simulink model "controller_observer_AllAgents_v5"  (Simulink 8.4) (continued)
== system controller_observer_AllAgents_v5/Controller == (continued)
  [TzCornerClass2Pod1] -> CornerClass2Pod1_Cooling_Mux/1
  [Tst_Cooling] -> CornerClass2Pod1_Cooling_Mux/5
  [Tamb] -> CornerClass2Pod1_Cooling_Mux/2
  CornerClass2Pod1_Cooling/1(Out1) -> [mcCornerClass2Pod1_Cooling]
  [TzNeighborCornerClass2Pod1] -> CornerClass2Pod1_Cooling_Mux/6
  CornerClass2Pod1_Cooling/3(Out3) -> [TstRefCornerClass2Pod1_Cooling]
  [TcCornerClass2Pod1_Cooling] -> CornerClass2Pod1_Cooling_Mux/3
  CornerClass2Pod1_Cooling_Mux/1 -> CornerClass2Pod1_Cooling/1(In1)
  [TsaCornerClass2Pod1] -> CornerClass2Pod1_Cooling_Mux/4
  CornerClass2Pod1_Cooling/2(Out2) -> [Mc_Tst_Tc_CornerClass2Pod1_Cooling]
  [TzCornerClass1Pod2] -> CornerClass1Pod2_Cooling_Mux/1
  [Tst_Cooling] -> CornerClass1Pod2_Cooling_Mux/5
  [Tamb] -> CornerClass1Pod2_Cooling_Mux/2
  CornerClass1Pod2_Cooling/1(Out1) -> [mcCornerClass1Pod2_Cooling]
  [TzNeighborCornerClass1Pod2] -> CornerClass1Pod2_Cooling_Mux/6
  CornerClass1Pod2_Cooling/3(Out3) -> [TstRefCornerClass1Pod2_Cooling]
  [TcCornerClass1Pod2_Cooling] -> CornerClass1Pod2_Cooling_Mux/3
  [TsaCornerClass1Pod2] -> CornerClass1Pod2_Cooling_Mux/4
  CornerClass1Pod2_Cooling/2(Out2) -> [Mc_Tst_Tc_CornerClass1Pod2_Cooling]
  [TzCornerClass2Pod2] -> CornerClass2Pod2_Cooling_Mux/1
  [Tst_Cooling] -> CornerClass2Pod2_Cooling_Mux/5
  [Tamb] -> CornerClass2Pod2_Cooling_Mux/2
  CornerClass2Pod2_Cooling/1(Out1) -> [mcCornerClass2Pod2_Cooling]
  [TzNeighborCornerClass2Pod2] -> CornerClass2Pod2_Cooling_Mux/6
  CornerClass2Pod2_Cooling/3(Out3) -> [TstRefCornerClass2Pod2_Cooling]
  [TcCornerClass2Pod2_Cooling] -> CornerClass2Pod2_Cooling_Mux/3
  CornerClass2Pod2_Cooling_Mux/1 -> CornerClass2Pod2_Cooling/1(In1)
  [TsaCornerClass2Pod2] -> CornerClass2Pod2_Cooling_Mux/4
  CornerClass2Pod2_Cooling/2(Out2) -> [Mc_Tst_Tc_CornerClass2Pod2_Cooling]
  [TzCornerClass1Pod3] -> CornerClass1Pod3_Cooling_Mux/1
  [Tst_Cooling] -> CornerClass1Pod3_Cooling_Mux/5
  [Tamb] -> CornerClass1Pod3_Cooling_Mux/2
  CornerClass1Pod3_Cooling/1(Out1) -> [mcCornerClass1Pod3_Cooling]
  [TzNeighborCornerClass1Pod3] -> CornerClass1Pod3_Cooling_Mux/6
  CornerClass1Pod3_Cooling/3(Out3) -> [TstRefCornerClass1Pod3_Cooling]
  [TcCornerClass1Pod3_Cooling] -> CornerClass1Pod3_Cooling_Mux/3
  CornerClass1Pod3_Cooling_Mux/1 -> CornerClass1Pod3_Cooling/1(In1)
  [TsaCornerClass1Pod3] -> CornerClass1Pod3_Cooling_Mux/4
  CornerClass1Pod3_Cooling/2(Out2) -> [Mc_Tst_Tc_CornerClass1Pod3_Cooling]
  [TzCornerClass2Pod3] -> CornerClass2Pod3_Cooling_Mux/1
  [Tst_Cooling] -> CornerClass2Pod3_Cooling_Mux/5
  [Tamb] -> CornerClass2Pod3_Cooling_Mux/2
  CornerClass2Pod3_Cooling/1(Out1) -> [mcCornerClass2Pod3_Cooling]
  [TzNeighborCornerClass2Pod3] -> CornerClass2Pod3_Cooling_Mux/6
  CornerClass2Pod3_Cooling/3(Out3) -> [TstRefCornerClass2Pod3_Cooling]
  [TcCornerClass2Pod3_Cooling] -> CornerClass2Pod3_Cooling_Mux/3
  CornerClass2Pod3_Cooling_Mux/1 -> CornerClass2Pod3_Cooling/1(In1)
  [TsaCornerClass2Pod3] -> CornerClass2Pod3_Cooling_Mux/4
  CornerClass2Pod3_Cooling/2(Out2) -> [Mc_Tst_Tc_CornerClass2Pod3_Cooling]
  [TzMultiClass1Pod1] -> MultiClass1Pod1_Cooling_Mux/1
  [Tst_Cooling] -> MultiClass1Pod1_Cooling_Mux/5
  [Tamb] -> MultiClass1Pod1_Cooling_Mux/2
  MultiClass1Pod1_Cooling/1(Out1) -> [mcMultiClass1Pod1_Cooling]
  [TzNeighborMultiClass1Pod1] -> MultiClass1Pod1_Cooling_Mux/6
  MultiClass1Pod1_Cooling/3(Out3) -> [TstRefMultiClass1Pod1_Cooling]
  [TcMultiClass1Pod1_Cooling] -> MultiClass1Pod1_Cooling_Mux/3
  MultiClass1Pod1_Cooling_Mux/1 -> MultiClass1Pod1_Cooling/1(In1)
  [TsaMultiClass1Pod1] -> MultiClass1Pod1_Cooling_Mux/4
  MultiClass1Pod1_Cooling/2(Out2) -> [Mc_Tst_Tc_MultiClass1Pod1_Cooling]
  [TzMultiClass2Pod1] -> MultiClass2Pod1_Cooling_Mux/1
  [Tst_Cooling] -> MultiClass2Pod1_Cooling_Mux/5
  [Tamb] -> MultiClass2Pod1_Cooling_Mux/2
  MultiClass2Pod1_Cooling/1(Out1) -> [mcMultiClass2Pod1_Cooling]
  [TzNeighborMultiClass2Pod1] -> MultiClass2Pod1_Cooling_Mux/6
  MultiClass2Pod1_Cooling/3(Out3) -> [TstRefMultiClass2Pod1_Cooling]
  [TcMultiClass2Pod1_Cooling] -> MultiClass2Pod1_Cooling_Mux/3
  MultiClass2Pod1_Cooling_Mux/1 -> MultiClass2Pod1_Cooling/1(In1)
  [TsaMultiClass2Pod1] -> MultiClass2Pod1_Cooling_Mux/4
  MultiClass2Pod1_Cooling/2(Out2) -> [Mc_Tst_Tc_MultiClass2Pod1_Cooling]
  [TzMultiClass1Pod2] -> MultiClass1Pod2_Cooling_Mux/1
  [Tst_Cooling] -> MultiClass1Pod2_Cooling_Mux/5
  [Tamb] -> MultiClass1Pod2_Cooling_Mux/2
  MultiClass1Pod2_Cooling/1(Out1) -> [mcMultiClass1Pod2_Cooling]
  [TzNeighborMultiClass1Pod2] -> MultiClass1Pod2_Cooling_Mux/6
  MultiClass1Pod2_Cooling/3(Out3) -> [TstRefMultiClass1Pod2_Cooling]
  [TcMultiClass1Pod2_Cooling] -> MultiClass1Pod2_Cooling_Mux/3
  MultiClass1Pod2_Cooling_Mux/1 -> MultiClass1Pod2_Cooling/1(In1)
  [TsaMultiClass1Pod2] -> MultiClass1Pod2_Cooling_Mux/4
  MultiClass1Pod2_Cooling/2(Out2) -> [Mc_Tst_Tc_MultiClass1Pod2_Cooling]
  [TzMultiClass2Pod2] -> MultiClass2Pod2_Cooling_Mux/1
  [Tst_Cooling] -> MultiClass2Pod2_Cooling_Mux/5
  [Tamb] -> MultiClass2Pod2_Cooling_Mux/2
  MultiClass2Pod2_Cooling/1(Out1) -> [mcMultiClass2Pod2_Cooling]
  [TzNeighborMultiClass2Pod2] -> MultiClass2Pod2_Cooling_Mux/6
  MultiClass2Pod2_Cooling/3(Out3) -> [TstRefMultiClass2Pod2_Cooling]
  [TcMultiClass2Pod2_Cooling] -> MultiClass2Pod2_Cooling_Mux/3
  MultiClass2Pod2_Cooling_Mux/1 -> MultiClass2Pod2_Cooling/1(In1)
  [TsaMultiClass2Pod2] -> MultiClass2Pod2_Cooling_Mux/4
  MultiClass2Pod2_Cooling/2(Out2) -> [Mc_Tst_Tc_MultiClass2Pod2_Cooling]
  [TzMultiClass1Pod3] -> MultiClass1Pod3_Cooling_Mux/1
  [Tst_Cooling] -> MultiClass1Pod3_Cooling_Mux/5
  [Tamb] -> MultiClass1Pod3_Cooling_Mux/2
  MultiClass1Pod3_Cooling/1(Out1) -> [mcMultiClass1Pod3_Cooling]
  [TzNeighborMultiClass1Pod3] -> MultiClass1Pod3_Cooling_Mux/6
  MultiClass1Pod3_Cooling/3(Out3) -> [TstRefMultiClass1Pod3_Cooling]
  [TcMultiClass1Pod3_Cooling] -> MultiClass1Pod3_Cooling_Mux/3
  MultiClass1Pod3_Cooling_Mux/1 -> MultiClass1Pod3_Cooling/1(In1)
  [TsaMultiClass1Pod3] -> MultiClass1Pod3_Cooling_Mux/4
  MultiClass1Pod3_Cooling/2(Out2) -> [Mc_Tst_Tc_MultiClass1Pod3_Cooling]
  [TzMultiClass2Pod3] -> MultiClass2Pod3_Cooling_Mux/1
  [Tst_Cooling] -> MultiClass2Pod3_Cooling_Mux/5
  [Tamb] -> MultiClass2Pod3_Cooling_Mux/2
  MultiClass2Pod3_Cooling/1(Out1) -> [mcMultiClass2Pod3_Cooling]
  [TzNeighborMultiClass2Pod3] -> MultiClass2Pod3_Cooling_Mux/6
  MultiClass2Pod3_Cooling/3(Out3) -> [TstRefMultiClass2Pod3_Cooling]
  [TcMultiClass2Pod3_Cooling] -> MultiClass2Pod3_Cooling_Mux/3
  MultiClass2Pod3_Cooling_Mux/1 -> MultiClass2Pod3_Cooling/1(In1)
  [TsaMultiClass2Pod3] -> MultiClass2Pod3_Cooling_Mux/4
  MultiClass2Pod3_Cooling/2(Out2) -> [Mc_Tst_Tc_MultiClass2Pod3_Cooling]
  [TzCorridorPod1] -> CorridorPod1_Cooling_Mux/1
  [Tst_Cooling] -> CorridorPod1_Cooling_Mux/5
  [Tamb] -> CorridorPod1_Cooling_Mux/2
  CorridorPod1_Cooling/1(Out1) -> [mcCorridorPod1_Cooling]
  [TzNeighborCorridorPod1] -> CorridorPod1_Cooling_Mux/6
  CorridorPod1_Cooling/3(Out3) -> [TstRefCorridorPod1_Cooling]
  [TcCorridorPod1_Cooling] -> CorridorPod1_Cooling_Mux/3
  CorridorPod1_Cooling_Mux/1 -> CorridorPod1_Cooling/1(In1)
  [TsaCorridorPod1] -> CorridorPod1_Cooling_Mux/4
  CorridorPod1_Cooling/2(Out2) -> [Mc_Tst_Tc_CorridorPod1_Cooling]
  [TzCorridorPod2] -> CorridorPod2_Cooling_Mux/1
  [Tst_Cooling] -> CorridorPod2_Cooling_Mux/5
  [Tamb] -> CorridorPod2_Cooling_Mux/2
  CorridorPod2_Cooling/1(Out1) -> [mcCorridorPod2_Cooling]
  [TzNeighborCorridorPod2] -> CorridorPod2_Cooling_Mux/6
  CorridorPod2_Cooling/3(Out3) -> [TstRefCorridorPod2_Cooling]
  [TcCorridorPod2_Cooling] -> CorridorPod2_Cooling_Mux/3
  CorridorPod2_Cooling_Mux/1 -> CorridorPod2_Cooling/1(In1)
  [TsaCorridorPod2] -> CorridorPod2_Cooling_Mux/4
  CorridorPod2_Cooling/2(Out2) -> [Mc_Tst_Tc_CorridorPod2_Cooling]
  [TzCorridorPod3] -> CorridorPod3_Cooling_Mux/1
  [Tst_Cooling] -> CorridorPod3_Cooling_Mux/5
  [Tamb] -> CorridorPod3_Cooling_Mux/2
  CorridorPod3_Cooling/1(Out1) -> [mcCorridorPod3_Cooling]
  [TzNeighborCorridorPod3] -> CorridorPod3_Cooling_Mux/6
  CorridorPod3_Cooling/3(Out3) -> [TstRefCorridorPod3_Cooling]
  [TcCorridorPod3_Cooling] -> CorridorPod3_Cooling_Mux/3
  CorridorPod3_Cooling_Mux/1 -> CorridorPod3_Cooling/1(In1)
  [TsaCorridorPod3] -> CorridorPod3_Cooling_Mux/4
  CorridorPod3_Cooling/2(Out2) -> [Mc_Tst_Tc_CorridorPod3_Cooling]
  [TzComputerClass] -> ComputerClass_Cooling_Mux/1
  [Tst_Cooling] -> ComputerClass_Cooling_Mux/5
  [Tamb] -> ComputerClass_Cooling_Mux/2
  ComputerClass_Cooling/1(Out1) -> [mcComputerClass_Cooling]
  [TzNeighborComputerClass] -> ComputerClass_Cooling_Mux/6
  ComputerClass_Cooling/3(Out3) -> [TstRefComputerClass_Cooling]
  [TcComputerClass_Cooling] -> ComputerClass_Cooling_Mux/3
  ComputerClass_Cooling_Mux/1 -> ComputerClass_Cooling/1(In1)
  [TsaComputerClass] -> ComputerClass_Cooling_Mux/4
  ComputerClass_Cooling/2(Out2) -> [Mc_Tst_Tc_ComputerClass_Cooling]
  [TzLibrary] -> LibraryMediaCenter_Cooling_Mux/1
  [Tst_Cooling] -> LibraryMediaCenter_Cooling_Mux/5
  [Tamb] -> LibraryMediaCenter_Cooling_Mux/2
  LibraryMediaCenter_Cooling/1(Out1) -> [mcLibrary_Cooling]
  [TzNeighborLibrary] -> LibraryMediaCenter_Cooling_Mux/6
  LibraryMediaCenter_Cooling/3(Out3) -> [TstRefLibrary_Cooling]
  [TcLibrary_Cooling] -> LibraryMediaCenter_Cooling_Mux/3
  LibraryMediaCenter_Cooling_Mux/1 -> LibraryMediaCenter_Cooling/1(In1)
  [TsaLibrary] -> LibraryMediaCenter_Cooling_Mux/4
  LibraryMediaCenter_Cooling/2(Out2) -> [Mc_Tst_Tc_Library_Cooling]
  [TzMainCorridor] -> MainCorridor_Cooling_Mux/1
  [Tst_Cooling] -> MainCorridor_Cooling_Mux/5
  [Tamb] -> MainCorridor_Cooling_Mux/2
  MainCorridor_Cooling/1(Out1) -> [mcMainCorridor_Cooling]
  [TzNeighborMainCorridor] -> MainCorridor_Cooling_Mux/6
  MainCorridor_Cooling/3(Out3) -> [TstRefMainCorridor_Cooling]
  [TcMainCorridor_Cooling] -> MainCorridor_Cooling_Mux/3
  MainCorridor_Cooling_Mux/1 -> MainCorridor_Cooling/1(In1)
  [TsaMainCorridor] -> MainCorridor_Cooling_Mux/4
  MainCorridor_Cooling/2(Out2) -> [Mc_Tst_Tc_MainCorridor_Cooling]
  [TzMech] -> Mech_Cooling_Mux/1
  [Tst_Cooling] -> Mech_Cooling_Mux/5
  [Tamb] -> Mech_Cooling_Mux/2
  Mech_Cooling/1(Out1) -> [mcMech_Cooling]
  [TzNeighborMech] -> Mech_Cooling_Mux/6
  Mech_Cooling/3(Out3) -> [TstRefMech_Cooling]
  [TcMech_Cooling] -> Mech_Cooling_Mux/3
  Mech_Cooling_Mux/1 -> Mech_Cooling/1(In1)
  [TsaMech] -> Mech_Cooling_Mux/4
  Mech_Cooling/2(Out2) -> [Mc_Tst_Tc_Mech_Cooling]
  [TzOffices] -> Offices_Cooling_Mux/1
  [Tst_Cooling] -> Offices_Cooling_Mux/5
  [Tamb] -> Offices_Cooling_Mux/2
  Offices_Cooling/1(Out1) -> [mcOffices_Cooling]
  [TzNeighborOffices] -> Offices_Cooling_Mux/6
  Offices_Cooling/3(Out3) -> [TstRefOffices_Cooling]
  [TcOffices_Cooling] -> Offices_Cooling_Mux/3
  Offices_Cooling_Mux/1 -> Offices_Cooling/1(In1)
  [TsaOffices] -> Offices_Cooling_Mux/4
  Offices_Cooling/2(Out2) -> [Mc_Tst_Tc_Offices_Cooling]
  [TzCafeteria] -> Cafeteria_Cooling_Mux/1
  [Tst_Cooling] -> Cafeteria_Cooling_Mux/5
  [Tamb] -> Cafeteria_Cooling_Mux/2
  Cafeteria_Cooling/1(Out1) -> [mcCafeteria_Cooling]
  [TzNeighborCafeteria] -> Cafeteria_Cooling_Mux/6
  Cafeteria_Cooling/3(Out3) -> [TstRefCafeteria_Cooling]
  [TcCafeteria_Cooling] -> Cafeteria_Cooling_Mux/3
  Cafeteria_Cooling_Mux/1 -> Cafeteria_Cooling/1(In1)
  [TsaCafeteria] -> Cafeteria_Cooling_Mux/4
  Cafeteria_Cooling/2(Out2) -> [Mc_Tst_Tc_Cafeteria_Cooling]
  [TzKitchen] -> Kitchen_Cooling_Mux/1
  [Tst_Cooling] -> Kitchen_Cooling_Mux/5
  [Tamb] -> Kitchen_Cooling_Mux/2
  Kitchen_Cooling/1(Out1) -> [mcKitchen_Cooling]
  [TzNeighborKitchen] -> Kitchen_Cooling_Mux/6
  Kitchen_Cooling/3(Out3) -> [TstRefKitchen_Cooling]
  [TcKitchen_Cooling] -> Kitchen_Cooling_Mux/3
  Kitchen_Cooling_Mux/1 -> Kitchen_Cooling/1(In1)
  [TsaKitchen] -> Kitchen_Cooling_Mux/4
  Kitchen_Cooling/2(Out2) -> [Mc_Tst_Tc_Kitchen_Cooling]
  [TzGym] -> Gym_Cooling_Mux/1
  [Tst_Cooling] -> Gym_Cooling_Mux/5
  [Tamb] -> Gym_Cooling_Mux/2
  Gym_Cooling/1(Out1) -> [mcGym_Cooling]
  [TzNeighborGym] -> Gym_Cooling_Mux/6
  Gym_Cooling/3(Out3) -> [TstRefGym_Cooling]
  [TcGym_Cooling] -> Gym_Cooling_Mux/3
  Gym_Cooling_Mux/1 -> Gym_Cooling/1(In1)
  [TsaGym] -> Gym_Cooling_Mux/4
  Gym_Cooling/2(Out2) -> [Mc_Tst_Tc_Gym_Cooling]
  [TzLobby] -> Lobby_Cooling_Mux/1
  [Tst_Cooling] -> Lobby_Cooling_Mux/5
  [Tamb] -> Lobby_Cooling_Mux/2
  Lobby_Cooling/1(Out1) -> [mcLobby_Cooling]
  [TzNeighborLobby] -> Lobby_Cooling_Mux/6
  Lobby_Cooling/3(Out3) -> [TstRefLobby_Cooling]
  [TcLobby_Cooling] -> Lobby_Cooling_Mux/3
  Lobby_Cooling_Mux/1 -> Lobby_Cooling/1(In1)
  [TsaLobby] -> Lobby_Cooling_Mux/4
  Lobby_Cooling/2(Out2) -> [Mc_Tst_Tc_Lobby_Cooling]
  [TzBath] -> Bath_Cooling_Mux/1
  [Tst_Cooling] -> Bath_Cooling_Mux/5
  [Tamb] -> Bath_Cooling_Mux/2
  Bath_Cooling/1(Out1) -> [mcBath_Cooling]
  [TzNeighborBath] -> Bath_Cooling_Mux/6
  Bath_Cooling/3(Out3) -> [TstRefBath_Cooling]
  [TcBath_Cooling] -> Bath_Cooling_Mux/3
  Bath_Cooling_Mux/1 -> Bath_Cooling/1(In1)
  [TsaBath] -> Bath_Cooling_Mux/4
  Bath_Cooling/2(Out2) -> [Mc_Tst_Tc_Bath_Cooling]
  Mux22/1 -> [TzNeighborCornerClass1Pod1]
  [TzMultiClass1Pod1] -> Mux22/1
  [TzCorridorPod1] -> Mux22/2
  Mux23/1 -> [TzNeighborCornerClass2Pod1]
  Mux24/1 -> [TzNeighborCornerClass1Pod2]
  [TzMultiClass1Pod2] -> Mux24/1
  [TzCorridorPod2] -> Mux24/2
  Mux25/1 -> [TzNeighborCornerClass2Pod2]
  Mux26/1 -> [TzNeighborCornerClass1Pod3]
  [TzMultiClass1Pod3] -> Mux26/1
  [TzCorridorPod3] -> Mux26/2
  Mux27/1 -> [TzNeighborCornerClass2Pod3]
  Mux34/1 -> [TzNeighborMultiClass1Pod1]
  Mux35/1 -> [TzNeighborMultiClass2Pod1]
  Mux36/1 -> [TzNeighborMultiClass1Pod2]
  Mux37/1 -> [TzNeighborMultiClass2Pod2]
  Mux38/1 -> [TzNeighborMultiClass1Pod3]
  [TzCorridorPod3] -> Mux38/2
  Mux39/1 -> [TzNeighborMultiClass2Pod3]
  Mux40/1 -> [TzNeighborCorridorPod1]
  [TzCornerClass1Pod1] -> Mux40/1
  [TzMultiClass2Pod1] -> Mux40/4
  Mux41/1 -> [TzNeighborCorridorPod2]
  [TzCornerClass1Pod2] -> Mux41/1
  [TzMultiClass2Pod2] -> Mux41/4
  Mux42/1 -> [TzNeighborCorridorPod3]
  [TzCornerClass1Pod3] -> Mux42/1
  Mux43/1 -> [TzNeighborMainCorridor]
  Mux44/1 -> [TzNeighborMech]
  Mux45/1 -> [TzNeighborComputerClass]
  Mux46/1 -> [TzNeighborLibrary]
  [TzComputerClass] -> Mux46/2
  [TzCorridorPod3] -> Mux46/3
  Mux47/1 -> [TzNeighborOffices]
  Mux48/1 -> [TzNeighborLobby]
  Mux49/1 -> [TzNeighborCafeteria]
  [TzKitchen] -> Mux49/1
  Mux50/1 -> [TzNeighborKitchen]
  [TzGym] -> Mux50/1
  Mux51/1 -> [TzNeighborGym]
  [TzOffices] -> Mux51/1
  Mux52/1 -> [TzNeighborBath]
  [TzMainCorridor] -> Mux52/1
  [TzMech] -> Mux52/2
  [TzLibrary] -> Mux52/4
  [TzCafeteria] -> Mux52/3
  From250/1 -> MinMax/2
  From251/1 -> MinMax/4
  From252/1 -> MinMax/3
  From254/1 -> MinMax/5
  From253/1 -> MinMax/6
  From255/1 -> MinMax/7
  From256/1 -> MinMax/8
  From258/1 -> MinMax/9
  From257/1 -> MinMax/10
  From260/1 -> MinMax/11
  From259/1 -> MinMax/12
  From261/1 -> MinMax/13
  From263/1 -> MinMax/14
  From262/1 -> MinMax/15
  From264/1 -> MinMax/16
  From265/1 -> MinMax/17
  From266/1 -> MinMax/18
  From268/1 -> MinMax/19
  From267/1 -> MinMax/20
  From269/1 -> MinMax/21
  From270/1 -> MinMax/22
  From272/1 -> MinMax/23
  From271/1 -> MinMax/24
  From273/1 -> MinMax/25
  From274/1 -> Add/1
  From275/1 -> Add/2
  From277/1 -> Add/3
  From276/1 -> Add/4
  From279/1 -> Add/5
  From278/1 -> Add/6
  From280/1 -> Add/7
  From281/1 -> Add/8
  From283/1 -> Add/9
  From282/1 -> Add/10
  From285/1 -> Add/11
  From284/1 -> Add/12
  From286/1 -> Add/13
  From288/1 -> Add/14
  From287/1 -> Add/15
  From289/1 -> Add/16
  From290/1 -> Add/17
  From291/1 -> Add/18
  From293/1 -> Add/19
  From292/1 -> Add/20
  From294/1 -> Add/21
  From295/1 -> Add/22
  From297/1 -> Add/23
  From296/1 -> Add/24
  From298/1 -> Add/25
  Gain1/1 -> Goto4/1
  [TzCornerClass1Pod1] -> CornerClass1Pod1_Heating_Mux/1
  [Tst_Heating] -> CornerClass1Pod1_Heating_Mux/5
  [Tamb] -> CornerClass1Pod1_Heating_Mux/2
  CornerClass1Pod1_Heating/1(Out1) -> [mcCornerClass1Pod1_Heating]
  [TzNeighborCornerClass1Pod1] -> CornerClass1Pod1_Heating_Mux/6
  CornerClass1Pod1_Heating/3(Out3) -> [TstRefCornerClass1Pod1_Heating]
  [TcCornerClass1Pod1_Heating] -> CornerClass1Pod1_Heating_Mux/3
  CornerClass1Pod1_Heating_Mux/1 -> CornerClass1Pod1_Heating/1(In1)
  [TsaCornerClass1Pod1] -> CornerClass1Pod1_Heating_Mux/4
  CornerClass1Pod1_Heating/2(Out2) -> [Mc_Tst_Tc_CornerClass1Pod1_Heating]
  [TzCornerClass2Pod1] -> CornerClass2Pod1_Heating_Mux/1
  [Tst_Heating] -> CornerClass2Pod1_Heating_Mux/5
  [Tamb] -> CornerClass2Pod1_Heating_Mux/2
  CornerClass2Pod1_Heating/1(Out1) -> [mcCornerClass2Pod1_Heating]
  [TzNeighborCornerClass2Pod1] -> CornerClass2Pod1_Heating_Mux/6
  CornerClass2Pod1_Heating/3(Out3) -> [TstRefCornerClass2Pod1_Heating]
  [TcCornerClass2Pod1_Heating] -> CornerClass2Pod1_Heating_Mux/3
  CornerClass2Pod1_Heating_Mux/1 -> CornerClass2Pod1_Heating/1(In1)
  [TsaCornerClass2Pod1] -> CornerClass2Pod1_Heating_Mux/4
  CornerClass2Pod1_Heating/2(Out2) -> [Mc_Tst_Tc_CornerClass2Pod1_Heating]
  [TzCornerClass1Pod2] -> CornerClass1Pod2_Heating_Mux/1
  [Tst_Heating] -> CornerClass1Pod2_Heating_Mux/5
  [Tamb] -> CornerClass1Pod2_Heating_Mux/2
  CornerClass1Pod2_Heating/1(Out1) -> [mcCornerClass1Pod2_Heating]
  [TzNeighborCornerClass1Pod2] -> CornerClass1Pod2_Heating_Mux/6
  CornerClass1Pod2_Heating/3(Out3) -> [TstRefCornerClass1Pod2_Heating]
  [TcCornerClass1Pod2_Heating] -> CornerClass1Pod2_Heating_Mux/3
  CornerClass1Pod2_Heating_Mux/1 -> CornerClass1Pod2_Heating/1(In1)
  [TsaCornerClass1Pod2] -> CornerClass1Pod2_Heating_Mux/4
  CornerClass1Pod2_Heating/2(Out2) -> [Mc_Tst_Tc_CornerClass1Pod2_Heating]
  [TzCornerClass2Pod2] -> CornerClass2Pod2_Heating_Mux/1
  [Tst_Heating] -> CornerClass2Pod2_Heating_Mux/5
  [Tamb] -> CornerClass2Pod2_Heating_Mux/2
  CornerClass2Pod2_Heating/1(Out1) -> [mcCornerClass2Pod2_Heating]
  [TzNeighborCornerClass2Pod2] -> CornerClass2Pod2_Heating_Mux/6
  CornerClass2Pod2_Heating/3(Out3) -> [TstRefCornerClass2Pod2_Heating]
  [TcCornerClass2Pod2_Heating] -> CornerClass2Pod2_Heating_Mux/3
  CornerClass2Pod2_Heating_Mux/1 -> CornerClass2Pod2_Heating/1(In1)
  [TsaCornerClass2Pod2] -> CornerClass2Pod2_Heating_Mux/4
  CornerClass2Pod2_Heating/2(Out2) -> [Mc_Tst_Tc_CornerClass2Pod2_Heating]
  [TzCornerClass1Pod3] -> CornerClass1Pod3_Heating_Mux/1
  [Tst_Heating] -> CornerClass1Pod3_Heating_Mux/5
  [Tamb] -> CornerClass1Pod3_Heating_Mux/2
  CornerClass1Pod3_Heating/1(Out1) -> [mcCornerClass1Pod3_Heating]
  [TzNeighborCornerClass1Pod3] -> CornerClass1Pod3_Heating_Mux/6
  CornerClass1Pod3_Heating/3(Out3) -> [TstRefCornerClass1Pod3_Heating]
  [TcCornerClass1Pod3_Heating] -> CornerClass1Pod3_Heating_Mux/3
  CornerClass1Pod3_Heating_Mux/1 -> CornerClass1Pod3_Heating/1(In1)
  [TsaCornerClass1Pod3] -> CornerClass1Pod3_Heating_Mux/4
  CornerClass1Pod3_Heating/2(Out2) -> [Mc_Tst_Tc_CornerClass1Pod3_Heating]
  [TzCornerClass2Pod3] -> CornerClass2Pod3_Heating_Mux/1
  [Tst_Heating] -> CornerClass2Pod3_Heating_Mux/5
  [Tamb] -> CornerClass2Pod3_Heating_Mux/2
  CornerClass2Pod3_Heating/1(Out1) -> [mcCornerClass2Pod3_Heating]
  [TzNeighborCornerClass2Pod3] -> CornerClass2Pod3_Heating_Mux/6
  CornerClass2Pod3_Heating/3(Out3) -> [TstRefCornerClass2Pod3_Heating]
  [TcCornerClass2Pod3_Heating] -> CornerClass2Pod3_Heating_Mux/3
  CornerClass2Pod3_Heating_Mux/1 -> CornerClass2Pod3_Heating/1(In1)
  [TsaCornerClass2Pod3] -> CornerClass2Pod3_Heating_Mux/4
  CornerClass2Pod3_Heating/2(Out2) -> [Mc_Tst_Tc_CornerClass2Pod3_Heating]
  [TzMultiClass1Pod1] -> MultiClass1Pod1_Heating_Mux/1
  [Tst_Heating] -> MultiClass1Pod1_Heating_Mux/5
  [Tamb] -> MultiClass1Pod1_Heating_Mux/2
  MultiClass1Pod1_Heating/1(Out1) -> [mcMultiClass1Pod1_Heating]
  [TzNeighborMultiClass1Pod1] -> MultiClass1Pod1_Heating_Mux/6
  MultiClass1Pod1_Heating/3(Out3) -> [TstRefMultiClass1Pod1_Heating]
  [TcMultiClass1Pod1_Heating] -> MultiClass1Pod1_Heating_Mux/3
  MultiClass1Pod1_Heating_Mux/1 -> MultiClass1Pod1_Heating/1(In1)
  [TsaMultiClass1Pod1] -> MultiClass1Pod1_Heating_Mux/4
  MultiClass1Pod1_Heating/2(Out2) -> [Mc_Tst_Tc_MultiClass1Pod1_Heating]
  [TzMultiClass2Pod1] -> MultiClass2Pod1_Heating_Mux/1
  [Tst_Heating] -> MultiClass2Pod1_Heating_Mux/5
  [Tamb] -> MultiClass2Pod1_Heating_Mux/2
  MultiClass2Pod1_Heating/1(Out1) -> [mcMultiClass2Pod1_Heating]
  [TzNeighborMultiClass2Pod1] -> MultiClass2Pod1_Heating_Mux/6
  MultiClass2Pod1_Heating/3(Out3) -> [TstRefMultiClass2Pod1_Heating]
  [TcMultiClass2Pod1_Heating] -> MultiClass2Pod1_Heating_Mux/3
  MultiClass2Pod1_Heating_Mux/1 -> MultiClass2Pod1_Heating/1(In1)
  [TsaMultiClass2Pod1] -> MultiClass2Pod1_Heating_Mux/4
  MultiClass2Pod1_Heating/2(Out2) -> [Mc_Tst_Tc_MultiClass2Pod1_Heating]
  [TzMultiClass1Pod2] -> MultiClass1Pod2_Heating_Mux/1
  [Tst_Heating] -> MultiClass1Pod2_Heating_Mux/5
  [Tamb] -> MultiClass1Pod2_Heating_Mux/2
  MultiClass1Pod2_Heating/1(Out1) -> [mcMultiClass1Pod2_Heating]
  [TzNeighborMultiClass1Pod2] -> MultiClass1Pod2_Heating_Mux/6
  MultiClass1Pod2_Heating/3(Out3) -> [TstRefMultiClass1Pod2_Heating]
  [TcMultiClass1Pod2_Heating] -> MultiClass1Pod2_Heating_Mux/3
  MultiClass1Pod2_Heating_Mux/1 -> MultiClass1Pod2_Heating/1(In1)
  [TsaMultiClass1Pod2] -> MultiClass1Pod2_Heating_Mux/4
  MultiClass1Pod2_Heating/2(Out2) -> [Mc_Tst_Tc_MultiClass1Pod2_Heating]
  [TzMultiClass2Pod2] -> MultiClass2Pod2_Heating_Mux/1
  [Tst_Heating] -> MultiClass2Pod2_Heating_Mux/5
  [Tamb] -> MultiClass2Pod2_Heating_Mux/2
  MultiClass2Pod2_Heating/1(Out1) -> [mcMultiClass2Pod2_Heating]
  [TzNeighborMultiClass2Pod2] -> MultiClass2Pod2_Heating_Mux/6
  MultiClass2Pod2_Heating/3(Out3) -> [TstRefMultiClass2Pod2_Heating]
  [TcMultiClass2Pod2_Heating] -> MultiClass2Pod2_Heating_Mux/3
  MultiClass2Pod2_Heating_Mux/1 -> MultiClass2Pod2_Heating/1(In1)
  [TsaMultiClass2Pod2] -> MultiClass2Pod2_Heating_Mux/4
  MultiClass2Pod2_Heating/2(Out2) -> [Mc_Tst_Tc_MultiClass2Pod2_Heating]
  [TzMultiClass1Pod3] -> MultiClass1Pod3_Heating_Mux/1
  [Tst_Heating] -> MultiClass1Pod3_Heating_Mux/5
  [Tamb] -> MultiClass1Pod3_Heating_Mux/2
  MultiClass1Pod3_Heating/1(Out1) -> [mcMultiClass1Pod3_Heating]
  [TzNeighborMultiClass1Pod3] -> MultiClass1Pod3_Heating_Mux/6
  MultiClass1Pod3_Heating/3(Out3) -> [TstRefMultiClass1Pod3_Heating]
  [TcMultiClass1Pod3_Heating] -> MultiClass1Pod3_Heating_Mux/3
  MultiClass1Pod3_Heating_Mux/1 -> MultiClass1Pod3_Heating/1(In1)
  [TsaMultiClass1Pod3] -> MultiClass1Pod3_Heating_Mux/4
  MultiClass1Pod3_Heating/2(Out2) -> [Mc_Tst_Tc_MultiClass1Pod3_Heating]
  [TzMultiClass2Pod3] -> MultiClass2Pod3_Heating_Mux/1
  [Tst_Heating] -> MultiClass2Pod3_Heating_Mux/5
  [Tamb] -> MultiClass2Pod3_Heating_Mux/2
  MultiClass2Pod3_Heating/1(Out1) -> [mcMultiClass2Pod3_Heating]
  [TzNeighborMultiClass2Pod3] -> MultiClass2Pod3_Heating_Mux/6
  MultiClass2Pod3_Heating/3(Out3) -> [TstRefMultiClass2Pod3_Heating]
  [TcMultiClass2Pod3_Heating] -> MultiClass2Pod3_Heating_Mux/3
  MultiClass2Pod3_Heating_Mux/1 -> MultiClass2Pod3_Heating/1(In1)
  [TsaMultiClass2Pod3] -> MultiClass2Pod3_Heating_Mux/4
  MultiClass2Pod3_Heating/2(Out2) -> [Mc_Tst_Tc_MultiClass2Pod3_Heating]
  [TzCorridorPod1] -> CorridorPod1_Heating_Mux/1
  [Tst_Heating] -> CorridorPod1_Heating_Mux/5
  [Tamb] -> CorridorPod1_Heating_Mux/2
  CorridorPod1_Heating/1(Out1) -> [mcCorridorPod1_Heating]
  [TzNeighborCorridorPod1] -> CorridorPod1_Heating_Mux/6
  CorridorPod1_Heating/3(Out3) -> [TstRefCorridorPod1_Heating]
  [TcCorridorPod1_Heating] -> CorridorPod1_Heating_Mux/3
  CorridorPod1_Heating_Mux/1 -> CorridorPod1_Heating/1(In1)
  [TsaCorridorPod1] -> CorridorPod1_Heating_Mux/4
  CorridorPod1_Heating/2(Out2) -> [Mc_Tst_Tc_CorridorPod1_Heating]
  [TzCorridorPod2] -> CorridorPod2_Heating_Mux/1
  [Tst_Heating] -> CorridorPod2_Heating_Mux/5
  [Tamb] -> CorridorPod2_Heating_Mux/2
  CorridorPod2_Heating/1(Out1) -> [mcCorridorPod2_Heating]
  [TzNeighborCorridorPod2] -> CorridorPod2_Heating_Mux/6
  CorridorPod2_Heating/3(Out3) -> [TstRefCorridorPod2_Heating]
  [TcCorridorPod2_Heating] -> CorridorPod2_Heating_Mux/3
  CorridorPod2_Heating_Mux/1 -> CorridorPod2_Heating/1(In1)
  [TsaCorridorPod2] -> CorridorPod2_Heating_Mux/4
  CorridorPod2_Heating/2(Out2) -> [Mc_Tst_Tc_CorridorPod2_Heating]
  [TzCorridorPod3] -> CorridorPod3_Heating_Mux/1
  [Tst_Heating] -> CorridorPod3_Heating_Mux/5
  [Tamb] -> CorridorPod3_Heating_Mux/2
  CorridorPod3_Heating/1(Out1) -> [mcCorridorPod3_Heating]
  [TzNeighborCorridorPod3] -> CorridorPod3_Heating_Mux/6
  CorridorPod3_Heating/3(Out3) -> [TstRefCorridorPod3_Heating]
  [TcCorridorPod3_Heating] -> CorridorPod3_Heating_Mux/3
  CorridorPod3_Heating_Mux/1 -> CorridorPod3_Heating/1(In1)
  [TsaCorridorPod3] -> CorridorPod3_Heating_Mux/4
  CorridorPod3_Heating/2(Out2) -> [Mc_Tst_Tc_CorridorPod3_Heating]
  [TzComputerClass] -> ComputerClass_Heating_Mux/1
  [Tst_Heating] -> ComputerClass_Heating_Mux/5
  [Tamb] -> ComputerClass_Heating_Mux/2
  ComputerClass_Heating/1(Out1) -> [mcComputerClass_Heating]
  [TzNeighborComputerClass] -> ComputerClass_Heating_Mux/6
  ComputerClass_Heating/3(Out3) -> [TstRefComputerClass_Heating]
  [TcComputerClass_Heating] -> ComputerClass_Heating_Mux/3
  ComputerClass_Heating_Mux/1 -> ComputerClass_Heating/1(In1)
  [TsaComputerClass] -> ComputerClass_Heating_Mux/4
  ComputerClass_Heating/2(Out2) -> [Mc_Tst_Tc_ComputerClass_Heating]
  [TzLibrary] -> LibraryMediaCenter_Heating_Mux/1
  [Tst_Heating] -> LibraryMediaCenter_Heating_Mux/5
  [Tamb] -> LibraryMediaCenter_Heating_Mux/2
  LibraryMediaCenter_Heating/1(Out1) -> [mcLibrary_Heating]
  [TzNeighborLibrary] -> LibraryMediaCenter_Heating_Mux/6
  LibraryMediaCenter_Heating/3(Out3) -> [TstRefLibrary_Heating]
  [TcLibrary_Heating] -> LibraryMediaCenter_Heating_Mux/3
  LibraryMediaCenter_Heating_Mux/1 -> LibraryMediaCenter_Heating/1(In1)
  [TsaLibrary] -> LibraryMediaCenter_Heating_Mux/4
  LibraryMediaCenter_Heating/2(Out2) -> [Mc_Tst_Tc_Library_Heating]
  [TzMainCorridor] -> MainCorridor_Heating_Mux/1
  [Tst_Heating] -> MainCorridor_Heating_Mux/5
  [Tamb] -> MainCorridor_Heating_Mux/2
  MainCorridor_Heating/1(Out1) -> [mcMainCorridor_Heating]
  [TzNeighborMainCorridor] -> MainCorridor_Heating_Mux/6
  MainCorridor_Heating/3(Out3) -> [TstRefMainCorridor_Heating]
  [TcMainCorridor_Heating] -> MainCorridor_Heating_Mux/3
  MainCorridor_Heating_Mux/1 -> MainCorridor_Heating/1(In1)
  [TsaMainCorridor] -> MainCorridor_Heating_Mux/4
  MainCorridor_Heating/2(Out2) -> [Mc_Tst_Tc_MainCorridor_Heating]
  [TzMech] -> Mech_Heating_Mux/1
  [Tst_Heating] -> Mech_Heating_Mux/5
  [Tamb] -> Mech_Heating_Mux/2
  Mech_Heating/1(Out1) -> [mcMech_Heating]
  [TzNeighborMech] -> Mech_Heating_Mux/6
  Mech_Heating/3(Out3) -> [TstRefMech_Heating]
  [TcMech_Heating] -> Mech_Heating_Mux/3
  Mech_Heating_Mux/1 -> Mech_Heating/1(In1)
  [TsaMech] -> Mech_Heating_Mux/4
  Mech_Heating/2(Out2) -> [Mc_Tst_Tc_Mech_Heating]
  [TzOffices] -> Offices_Heating_Mux/1
  [Tst_Heating] -> Offices_Heating_Mux/5
  [Tamb] -> Offices_Heating_Mux/2
  Offices_Heating/1(Out1) -> [mcOffices_Heating]
  [TzNeighborOffices] -> Offices_Heating_Mux/6
  Offices_Heating/3(Out3) -> [TstRefOffices_Heating]
  [TcOffices_Heating] -> Offices_Heating_Mux/3
  Offices_Heating_Mux/1 -> Offices_Heating/1(In1)
  [TsaOffices] -> Offices_Heating_Mux/4
  Offices_Heating/2(Out2) -> [Mc_Tst_Tc_Offices_Heating]
  [TzCafeteria] -> Cafeteria_Heating_Mux/1
  [Tst_Heating] -> Cafeteria_Heating_Mux/5
  [Tamb] -> Cafeteria_Heating_Mux/2
  Cafeteria_Heating/1(Out1) -> [mcCafeteria_Heating]
  [TzNeighborCafeteria] -> Cafeteria_Heating_Mux/6
  Cafeteria_Heating/3(Out3) -> [TstRefCafeteria_Heating]
  [TcCafeteria_Heating] -> Cafeteria_Heating_Mux/3
  Cafeteria_Heating_Mux/1 -> Cafeteria_Heating/1(In1)
  [TsaCafeteria] -> Cafeteria_Heating_Mux/4
  Cafeteria_Heating/2(Out2) -> [Mc_Tst_Tc_Cafeteria_Heating]
  [TzKitchen] -> Kitchen_Heating_Mux/1
  [Tst_Heating] -> Kitchen_Heating_Mux/5
  [Tamb] -> Kitchen_Heating_Mux/2
  Kitchen_Heating/1(Out1) -> [mcKitchen_Heating]
  [TzNeighborKitchen] -> Kitchen_Heating_Mux/6
  Kitchen_Heating/3(Out3) -> [TstRefKitchen_Heating]
  [TcKitchen_Heating] -> Kitchen_Heating_Mux/3
  Kitchen_Heating_Mux/1 -> Kitchen_Heating/1(In1)
  [TsaKitchen] -> Kitchen_Heating_Mux/4
  Kitchen_Heating/2(Out2) -> [Mc_Tst_Tc_Kitchen_Heating]
  [TzGym] -> Gym_Heating_Mux/1
  [Tst_Heating] -> Gym_Heating_Mux/5
  [Tamb] -> Gym_Heating_Mux/2
  Gym_Heating/1(Out1) -> [mcGym_Heating]
  [TzNeighborGym] -> Gym_Heating_Mux/6
  Gym_Heating/3(Out3) -> [TstRefGym_Heating]
  [TcGym_Heating] -> Gym_Heating_Mux/3
  Gym_Heating_Mux/1 -> Gym_Heating/1(In1)
  [TsaGym] -> Gym_Heating_Mux/4
  Gym_Heating/2(Out2) -> [Mc_Tst_Tc_Gym_Heating]
  [TzLobby] -> Lobby_Heating_Mux/1
  [Tst_Heating] -> Lobby_Heating_Mux/5
  [Tamb] -> Lobby_Heating_Mux/2
  Lobby_Heating/1(Out1) -> [mcLobby_Heating]
  [TzNeighborLobby] -> Lobby_Heating_Mux/6
  Lobby_Heating/3(Out3) -> [TstRefLobby_Heating]
  [TcLobby_Heating] -> Lobby_Heating_Mux/3
  Lobby_Heating_Mux/1 -> Lobby_Heating/1(In1)
  [TsaLobby] -> Lobby_Heating_Mux/4
  Lobby_Heating/2(Out2) -> [Mc_Tst_Tc_Lobby_Heating]
  [TzBath] -> Bath_Heating_Mux/1
  [Tst_Heating] -> Bath_Heating_Mux/5
  [Tamb] -> Bath_Heating_Mux/2
  Bath_Heating/1(Out1) -> [mcBath_Heating]
  [TzNeighborBath] -> Bath_Heating_Mux/6
  Bath_Heating/3(Out3) -> [TstRefBath_Heating]
  [TcBath_Heating] -> Bath_Heating_Mux/3
  Bath_Heating_Mux/1 -> Bath_Heating/1(In1)
  [TsaBath] -> Bath_Heating_Mux/4
  Bath_Heating/2(Out2) -> [Mc_Tst_Tc_Bath_Heating]
  [mcCornerClass1Pod1_Heating] -> Fault_mc_CornerClass1Pod1_Heating/1(In)
  [mcCornerClass1Pod1_Cooling] -> Fault_mc_CornerClass1Pod1_Cooling/1(In)
  [mcCornerClass2Pod1_Cooling] -> Fault_mc_CornerClass2Pod1_Cooling/1(In)
  [mcCornerClass1Pod2_Cooling] -> Fault_mc_CornerClass1Pod2_Cooling/1(In)
  [mcCornerClass2Pod2_Cooling] -> Fault_mc_CornerClass2Pod2_Cooling/1(In)
  [mcCornerClass1Pod3_Cooling] -> Fault_mc_CornerClass1Pod3_Cooling/1(In)
  [mcCornerClass2Pod3_Cooling] -> Fault_mc_CornerClass2Pod3_Cooling/1(In)
  [mcMultiClass1Pod1_Cooling] -> Fault_mc_MultiClass1Pod1_Cooling/1(In)
  [mcMultiClass2Pod1_Cooling] -> Fault_mc_MultiClass2Pod1_Cooling/1(In)
  [mcMultiClass1Pod2_Cooling] -> Fault_mc_MultiClass1Pod2_Cooling/1(In)
  [mcMultiClass2Pod2_Cooling] -> Fault_mc_MultiClass2Pod2_Cooling/1(In)
  [mcMultiClass1Pod3_Cooling] -> Fault_mc_MultiClass1Pod3_Cooling/1(In)
  [mcMultiClass2Pod3_Cooling] -> Fault_mc_MultiClass2Pod3_Cooling/1(In)
  [mcCorridorPod1_Cooling] -> Fault_mc_CorridorPod1_Cooling/1(In)
  [mcCorridorPod2_Cooling] -> Fault_mc_CorridorPod2_Cooling/1(In)
  [mcCorridorPod3_Cooling] -> Fault_mc_CorridorPod3_Cooling/1(In)
  [mcMainCorridor_Cooling] -> Fault_mc_MainCorridor_Cooling/1(In)
  [mcMech_Cooling] -> Fault_mc_Mech_Cooling/1(In)
  [mcComputerClass_Cooling] -> Fault_mc_ComputerClass_Cooling/1(In)
  [mcLibrary_Cooling] -> Fault_mc_LibraryMediaCenter_Cooling/1(In)
  [mcOffices_Cooling] -> Fault_mc_Offices_Cooling/1(In)
  [mcLobby_Cooling] -> Fault_mc_Lobby_Cooling/1(In)
  [mcCafeteria_Cooling] -> Fault_mc_Cafeteria_Cooling/1(In)
  [mcKitchen_Cooling] -> Fault_mc_Kitchen_Cooling/1(In)
  [mcGym_Cooling] -> Fault_mc_Gym_Cooling/1(In)
  [mcBath_Cooling] -> Fault_mc_Bath_Cooling/1(In)
  [mcCornerClass2Pod1_Heating] -> Fault_mc_CornerClass2Pod1_Heating/1(In)
  [mcCornerClass1Pod2_Heating] -> Fault_mc_CornerClass1Pod2_Heating/1(In)
  [mcCornerClass2Pod2_Heating] -> Fault_mc_CornerClass2Pod2_Heating/1(In)
  [mcCornerClass1Pod3_Heating] -> Fault_mc_CornerClass1Pod3_Heating/1(In)
  [mcCornerClass2Pod3_Heating] -> Fault_mc_CornerClass2Pod3_Heating/1(In)
  [mcMultiClass1Pod1_Heating] -> Fault_mc_MultiClass1Pod1_Heating/1(In)
  [mcMultiClass2Pod1_Heating] -> Fault_mc_MultiClass2Pod1_Heating/1(In)
  [mcMultiClass1Pod2_Heating] -> Fault_mc_MultiClass1Pod2_Heating/1(In)
  [mcMultiClass2Pod2_Heating] -> Fault_mc_MultiClass2Pod2_Heating/1(In)
  [mcMultiClass1Pod3_Heating] -> Fault_mc_MultiClass1Pod3_Heating/1(In)
  [mcMultiClass2Pod3_Heating] -> Fault_mc_MultiClass2Pod3_Heating/1(In)
  [mcCorridorPod1_Heating] -> Fault_mc_CorridorPod1_Heating/1(In)
  [mcCorridorPod2_Heating] -> Fault_mc_CorridorPod2_Heating/1(In)
  [mcCorridorPod3_Heating] -> Fault_mc_CorridorPod3_Heating/1(In)
  [mcMainCorridor_Heating] -> Fault_mc_MainCorridor_Heating/1(In)
  [mcMech_Heating] -> Fault_mc_Mech_Heating/1(In)
  [mcComputerClass_Heating] -> Fault_mc_ComputerClass_Heating/1(In)
  [mcLibrary_Heating] -> Fault_mc_LibraryMediaCenter_Heating/1(In)
  [mcOffices_Heating] -> Fault_mc_Offices_Heating/1(In)
  [mcLobby_Heating] -> Fault_mc_Lobby_Heating/1(In)
  [mcCafeteria_Heating] -> Fault_mc_Cafeteria_Heating/1(In)
  [mcKitchen_Heating] -> Fault_mc_Kitchen_Heating/1(In)
  [mcGym_Heating] -> Fault_mc_Gym_Heating/1(In)
  [mcBath_Heating] -> Fault_mc_Bath_Heating/1(In)
  Demux/12 -> Fault_Tc_CornerClass1Pod2_Heating/1(In)
  Demux/20 -> Fault_Tc_CornerClass1Pod3_Heating/1(In)
  Demux/21 -> Fault_Tz_CornerClass2Pod3/1(In)
  Demux/40 -> Fault_Tc_MultiClass2Pod2_Heating/1(In)
  Demux/44 -> Fault_Tc_MultiClass1Pod3_Heating/1(In)
  Demux/56 -> Fault_Tc_CorridorPod2_Heating/1(In)
  Demux/61 -> Fault_Tz_MainCorridor/1(In)
  Demux/73 -> Fault_Tz_LibraryMediaCenter/1(In)
  Demux/88 -> Fault_Tc_Cafeteria_Heating/1(In)
  Demux/93 -> Fault_Tz_Gym/1(In)
  Demux/102 -> Fault_Tst_Cooling/1(In)
  Demux/103 -> Fault_Tst_Heating/1(In)
  Demux/1 -> Fault_Tz_CornerClass1Pod1/1(In)
  Demux/2 -> Fault_Tsa_CornerClass1Pod1/1(In)
  Demux/3 -> Fault_Tc_CornerClass1Pod1_Cooling/1(In)
  Demux/4 -> Fault_Tc_CornerClass1Pod1_Heating/1(In)
  Demux/5 -> Fault_Tz_CornerClass2Pod1/1(In)
  Demux/6 -> Fault_Tsa_CornerClass2Pod1/1(In)
  Demux/7 -> Fault_Tc_CornerClass2Pod1_Cooling/1(In)
  Demux/8 -> Fault_Tc_CornerClass2Pod1_Heating/1(In)
  Demux/9 -> Fault_Tz_CornerClass1Pod2/1(In)
  Demux/10 -> Fault_Tsa_CornerClass1Pod2/1(In)
  Demux/11 -> Fault_Tc_CornerClass1Pod2_Cooling/1(In)
  Demux/13 -> Fault_Tz_CornerClass2Pod2/1(In)
  Demux/14 -> Fault_Tsa_CornerClass2Pod2/1(In)
  Demux/15 -> Fault_Tc_CornerClass2Pod2_Cooling/1(In)
  Demux/16 -> Fault_Tc_CornerClass2Pod2_Heating/1(In)
  Demux/17 -> Fault_Tz_CornerClass1Pod3/1(In)
  Demux/18 -> Fault_Tsa_CornerClass1Pod3/1(In)
  Demux/19 -> Fault_Tc_CornerClass1Pod3_Cooling/1(In)
  Demux/22 -> Fault_Tsa_CornerClass2Pod3/1(In)
  Demux/23 -> Fault_Tc_CornerClass2Pod3_Cooling/1(In)
  Demux/24 -> Fault_Tc_CornerClass2Pod3_Heating/1(In)
  Demux/25 -> Fault_Tz_MultiClass1Pod1/1(In)
  Demux/26 -> Fault_Tsa_MultiClass1Pod1/1(In)
  Demux/27 -> Fault_Tc_MultiClass1Pod1_Cooling/1(In)
  Demux/28 -> Fault_Tc_MultiClass1Pod1_Heating/1(In)
  Demux/29 -> Fault_Tz_MultiClass2Pod1/1(In)
  Demux/30 -> Fault_Tsa_MultiClass2Pod1/1(In)
  Demux/31 -> Fault_Tc_MultiClass2Pod1_Cooling/1(In)
  Demux/32 -> Fault_Tc_MultiClass2Pod1_Heating/1(In)
  Demux/33 -> Fault_Tz_MultiClass1Pod2/1(In)
  Demux/34 -> Fault_Tsa_MultiClass1Pod2/1(In)
  Demux/35 -> Fault_Tc_MultiClass1Pod2_Cooling/1(In)
  Demux/36 -> Fault_Tc_MultiClass1Pod2_Heating/1(In)
  Demux/37 -> Fault_Tz_MultiClass2Pod2/1(In)
  Demux/38 -> Fault_Tsa_MultiClass2Pod2/1(In)
  Demux/39 -> Fault_Tc_MultiClass2Pod2_Cooling/1(In)
  Demux/41 -> Fault_Tz_MultiClass1Pod3/1(In)
  Demux/42 -> Fault_Tsa_MultiClass1Pod3/1(In)
  Demux/43 -> Fault_Tc_MultiClass1Pod3_Cooling/1(In)
  Demux/45 -> Fault_Tz_MultiClass2Pod3/1(In)
  Demux/46 -> Fault_Tsa_MultiClass2Pod3/1(In)
  Demux/47 -> Fault_Tc_MultiClass2Pod3_Cooling/1(In)
  Demux/48 -> Fault_Tc_MultiClass2Pod3_Heating/1(In)
  Demux/49 -> Fault_Tz_CorridorPod1/1(In)
  Demux/50 -> Fault_Tsa_CorridorPod1/1(In)
  Demux/51 -> Fault_Tc_CorridorPod1_Cooling/1(In)
  Demux/52 -> Fault_Tc_CorridorPod1_Heating/1(In)
  Demux/53 -> Fault_Tz_CorridorPod2/1(In)
  Demux/54 -> Fault_Tsa_CorridorPod2/1(In)
  Demux/55 -> Fault_Tc_CorridorPod2_Cooling/1(In)
  Demux/57 -> Fault_Tz_CorridorPod3/1(In)
  Demux/58 -> Fault_Tsa_CorridorPod3/1(In)
  Demux/59 -> Fault_Tc_CorridorPod3_Cooling/1(In)
  Demux/60 -> Fault_Tc_CorridorPod3_Heating/1(In)
  Demux/62 -> Fault_Tsa_MainCorridor/1(In)
  Demux/63 -> Fault_Tc_MainCorridor_Cooling/1(In)
  Demux/64 -> Fault_Tc_MainCorridor_Heating/1(In)
  Demux/65 -> Fault_Tz_Mech/1(In)
  Demux/66 -> Fault_Tsa_Mech/1(In)
  Demux/67 -> Fault_Tc_Mech_Cooling/1(In)
  Demux/68 -> Fault_Tc_Mech_Heating/1(In)
  Demux/69 -> Fault_Tz_ComputerClass/1(In)
  Demux/70 -> Fault_Tsa_ComputerClass/1(In)
  Demux/71 -> Fault_Tc_ComputerClass_Cooling/1(In)
  Demux/72 -> Fault_Tc_ComputerClass_Heating/1(In)
  Demux/74 -> Fault_Tsa_LibraryMediaCenter/1(In)
  Demux/75 -> Fault_Tc_LibraryMediaCenter_Cooling/1(In)
  Demux/76 -> Fault_Tc_LibraryMediaCenter_Heating/1(In)
  Demux/77 -> Fault_Tz_Offices/1(In)
  Demux/78 -> Fault_Tsa_Offices/1(In)
  Demux/79 -> Fault_Tc_Offices_Cooling/1(In)
  Demux/80 -> Fault_Tc_Offices_Heating/1(In)
  Demux/81 -> Fault_Tz_Lobby/1(In)
  Demux/82 -> Fault_Tsa_Lobby/1(In)
  Demux/83 -> Fault_Tc_Lobby_Cooling/1(In)
  Demux/84 -> Fault_Tc_Lobby_Heating/1(In)
  Demux/85 -> Fault_Tz_Cafeteria/1(In)
  Demux/86 -> Fault_Tsa_Cafeteria/1(In)
  Demux/87 -> Fault_Tc_Cafeteria_Cooling/1(In)
  Demux/89 -> Fault_Tz_Kitchen/1(In)
  Demux/90 -> Fault_Tsa_Kitchen/1(In)
  Demux/91 -> Fault_Tc_Kitchen_Cooling/1(In)
  Demux/92 -> Fault_Tc_Kitchen_Heating/1(In)
  Demux/94 -> Fault_Tsa_Gym/1(In)
  Demux/95 -> Fault_Tc_Gym_Cooling/1(In)
  Demux/96 -> Fault_Tc_Gym_Heating/1(In)
  Demux/97 -> Fault_Tz_Bath/1(In)
  Demux/98 -> Fault_Tsa_Bath/1(In)
  Demux/99 -> Fault_Tc_Bath_Cooling/1(In)
  Demux/100 -> Fault_Tc_Bath_Heating/1(In)
  Demux/101 -> Fault_Tamb/1(In)
  [TzCorridorPod1] -> Mux23/1
  [TzMultiClass2Pod1] -> Mux23/2
  [TzCorridorPod2] -> Mux25/1
  [TzMultiClass2Pod2] -> Mux25/2
  [TzCorridorPod3] -> Mux27/1
  [TzMultiClass2Pod3] -> Mux27/2
  [TzCornerClass1Pod1] -> Mux34/3
  [TzCorridorPod1] -> Mux34/2
  [TzLobby] -> Mux34/1
  [TzCorridorPod1] -> Mux35/1
  [TzMainCorridor] -> Mux35/2
  [TzCornerClass2Pod1] -> Mux35/3
  [TzCorridorPod2] -> Mux36/2
  [TzCornerClass1Pod2] -> Mux36/3
  [TzMainCorridor] -> Mux36/1
  [TzCorridorPod2] -> Mux37/1
  [TzMainCorridor] -> Mux37/2
  [TzCornerClass2Pod2] -> Mux37/3
  [TzLibrary] -> Mux38/1
  [TzCornerClass1Pod3] -> Mux38/3
  [TzCorridorPod3] -> Mux39/1
  [TzComputerClass] -> Mux39/2
  [TzCornerClass2Pod3] -> Mux39/3
  [TzMainCorridor] -> Mux40/3
  [TzCornerClass2Pod1] -> Mux40/5
  [TzMultiClass1Pod1] -> Mux40/2
  [TzMultiClass1Pod2] -> Mux41/2
  [TzMainCorridor] -> Mux41/3
  [TzCornerClass2Pod2] -> Mux41/5
  [TzMultiClass1Pod3] -> Mux42/2
  [TzLibrary] -> Mux42/3
  [TzComputerClass] -> Mux42/4
  [TzMultiClass2Pod3] -> Mux42/5
  [TzCornerClass2Pod3] -> Mux42/6
  [TzBath] -> Mux43/3
  [TzMech] -> Mux43/2
  [TzLobby] -> Mux43/1
  [TzMultiClass2Pod2] -> Mux43/4
  [TzCorridorPod2] -> Mux43/5
  [TzMultiClass1Pod2] -> Mux43/6
  [TzCorridorPod1] -> Mux43/8
  [TzMultiClass2Pod1] -> Mux43/7
  [TzLobby] -> Mux44/1
  [TzOffices] -> Mux44/2
  [TzGym] -> Mux44/3
  [TzKitchen] -> Mux44/4
  [TzCafeteria] -> Mux44/5
  [TzMainCorridor] -> Mux44/7
  [TzBath] -> Mux44/6
  [TzCorridorPod3] -> Mux45/1
  [TzLibrary] -> Mux45/2
  [TzMultiClass2Pod3] -> Mux45/3
  [TzMultiClass1Pod3] -> Mux46/4
  [TzBath] -> Mux46/1
  [TzLobby] -> Mux47/3
  [TzGym] -> Mux47/1
  [TzMech] -> Mux47/2
  [TzMainCorridor] -> Mux48/3
  [TzMultiClass1Pod1] -> Mux48/4
  [TzMech] -> Mux48/2
  [TzOffices] -> Mux48/1
  [TzBath] -> Mux49/2
  [TzMech] -> Mux49/3
  [TzCafeteria] -> Mux50/2
  [TzMech] -> Mux50/3
  [TzKitchen] -> Mux51/2
  [TzMech] -> Mux51/3
  Mux2/1 -> Saturation/1
  [On_Off] -> CornerClass1Pod1_Cooling/Enable
  [On_Off] -> CornerClass1Pod1_Heating/Enable